simulink model "v01_16r4t11f_ver149bb"  (Simulink 7.9) (continued)
== system v01_16r4t11f_ver149bb == (continued)
      mask init: same as v01_16r4t11f_ver149bb/adcsnap0
      mask StyleString: popup(bram|dram),popup(1|2|3|4),popup(200|250|266|300|333),edit,popup(8|16|32|64|128),checkbox,checkbox,checkbox,checkbox
      mask CallbackString: snapshot_callback();||||||||
  sg : Subsystem
  speader1 : Subsystem  mask{spead_msw=64, spead_lsw=40, num_items=6, num_payloads=2}
      mask prompts: num_items "Number of items (+5)"; num_payloads "number of payloads"
      mask help:
      | The size of the heap and payload are in Bytes.
      | If there is one packet per heap then payload_offset is 0.
      | All the input must be not longer than 40 bits.
  spectrum_cntr : xbsIndex_r4/Counter  cnt_type=Free Running  cnt_to=Inf  operation=Up  start_count=0  cnt_by_val=1  arith_type=Unsigned  n_bits=40  bin_pt=0  load_pin=off  rst=on  en=on  explicit_period=on  period=1  dbl_ovrd=off  use_behavioral_HDL=off  implementation=Fabric  block_type=counter
      settings: block_version=11.4
  ssg : Subsystem
  status : xps_library/software register  Tag=xps:sw_reg  io_dir=To Processor  arith_type=Unsigned  bitwidth=32  bin_pt=0  sample_period=1  gw_name=v01_16r4t11f_ver148bb_status_user_data_in
  stokesout : Subsystem  AncestorBlock=casper_library_scopes/snapshot  mask(snapshot){storage=bram, dram_dimm=1, dram_clock=200, nsamples=10, data_width=32, offset=off, circap=off, value=off, use_dsp48=on}
      mask prompts: storage "Storage medium"; dram_dimm "DRAM dimm"; dram_clock "DRAM clock rate"; nsamples "Number of Samples (2^?)"; data_width "Data width"; offset "Start delay support"; circap "Circular capture support"; value "Extra value capture support"; use_dsp48 "Use DSP48s to implement counters"
      mask help:
      | Standard snap block, but with the following optional extras;
      |  1) Optionally delay the fabric trigger by a user-specified number of valids.
      |  2) Capture continuously after a trigger until a stop pulse is received. Data is captured into BRAM continuously, with old data being overwritten until a stop command is received. Then stop capturing when memory is filled. This enables you to get some data before and some data after the stop pulse. Use the 'tr_en_cnt' register to determine where you are in your vector. 'Addr' register's MSb now indicates 'busy capturing'.
      |  3) Optionally capture a value on an input port to a register at the same time as the first data sample.
      |  4) Implement counters using DSP48Es to save resources.
      mask init: same as v01_16r4t11f_ver149bb/adcsnap0
      mask StyleString: popup(bram|dram),popup(1|2|3|4),popup(200|250|266|300|333),edit,popup(8|16|32|64|128),checkbox,checkbox,checkbox,checkbox
      mask CallbackString: snapshot_callback();||||||||
  sync_delay : casper_library_delays/sync_delay  DelayLen=760*128
  time_cntr : xbsIndex_r4/Counter  cnt_type=Free Running  cnt_to=Inf  operation=Up  start_count=0  cnt_by_val=1  arith_type=Unsigned  n_bits=40  bin_pt=0  load_pin=off  rst=on  en=off  explicit_period=on  period=1  dbl_ovrd=off  use_behavioral_HDL=off  implementation=Fabric  block_type=counter
      settings: block_version=11.4
  timesel : xps_library/software register  Tag=xps:sw_reg  io_dir=From Processor  arith_type=Unsigned  bitwidth=32  bin_pt=0  sample_period=1  gw_name=v01_16r4t11f_ver148bb_timesel_user_data_out
  timesnap : Subsystem  AncestorBlock=casper_library_scopes/snapshot  mask(snapshot){storage=bram, dram_dimm=1, dram_clock=200, nsamples=10, data_width=64, offset=off, circap=off, value=off, use_dsp48=on}
      mask prompts: storage "Storage medium"; dram_dimm "DRAM dimm"; dram_clock "DRAM clock rate"; nsamples "Number of Samples (2^?)"; data_width "Data width"; offset "Start delay support"; circap "Circular capture support"; value "Extra value capture support"; use_dsp48 "Use DSP48s to implement counters"
      mask help:
      | Standard snap block, but with the following optional extras;
      |  1) Optionally delay the fabric trigger by a user-specified number of valids.
      |  2) Capture continuously after a trigger until a stop pulse is received. Data is captured into BRAM continuously, with old data being overwritten until a stop command is received. Then stop capturing when memory is filled. This enables you to get some data before and some data after the stop pulse. Use the 'tr_en_cnt' register to determine where you are in your vector. 'Addr' register's MSb now indicates 'busy capturing'.
      |  3) Optionally capture a value on an input port to a register at the same time as the first data sample.
      |  4) Implement counters using DSP48Es to save resources.
      mask init: same as v01_16r4t11f_ver149bb/adcsnap0
      mask StyleString: popup(bram|dram),popup(1|2|3|4),popup(200|250|266|300|333),edit,popup(8|16|32|64|128),checkbox,checkbox,checkbox,checkbox
      mask CallbackString: snapshot_callback();||||||||
  vacc0 : Subsystem  AncestorBlock=gavrt_library/vacc  mask(vacc){veclen=7, n_inputs=4, max_accum=10, arith_type=1, in_bit_width=32, in_bin_pt=0, out_bit_width=42, out_bin_pt=0, register_din=1, add_latency=2, bram_latency=2, mux_latency=0, use_dsp48=1}
      mask prompts: veclen "Vector Length (2^?)"; n_inputs "Number of Simultaneous Vectors"; max_accum "Maximum # Accumulations (2^?)"; arith_type "Arithmetic Type (0=Unsigned, 1=Signed)"; in_bit_width "Input Bit Width"; in_bin_pt "Input Binary Point"; out_bit_width "Output Bit Width"; out_bin_pt "Output Binary Point"; register_din "Register Input Data  (1 = Enable Register Retiming on input delay)"; add_latency "Add Latency"; bram_latency "BRAM Latency"; mux_latency "Mux Latency"; use_dsp48 "Use DSP48"
      mask help:
      | Sync is expected the clock before the first data to be accumulated.
      | Use the Cram block to feed din for multiple simultaneous inputs.
      | acc_len is the number of vectors to sum.
      | valid is high while a valid accumulation is being clocked out on dout.
      | If only one sync pulse is provided, valid accumulations will repeatedly appear on dout as they become available.
      | Undesired behavior may occur if acc_len is changed without a new sync pulse.
      | Note: The output is an unsigned concatenation of the output streams. Thus, even with only one stream, the output must be recast before using.
      | Register Input Data controls the "Enable Register Retiming" parameter of the delays on 'din'.
      mask init:
      | config.toplevel = gcb;
      | config.source = str2func('vacc_init_xblock');
      | xBlock( config, {...
      |     'veclen', veclen, ...
      |     'n_inputs', n_inputs, ...
      |     'max_accum', max_accum, ...
      |     'arith_type', arith_type, ...
      |     'in_bit_width', in_bit_width, ...
      |     'in_bin_pt', in_bin_pt, ...
      |     'out_bit_width', out_bit_width, ...
      |     'out_bin_pt', out_bin_pt, ...
      |     'register_din', register_din, ...
      |     'add_latency', add_latency, ...
      |     'bram_latency', bram_latency, ...
      |     'mux_latency', mux_latency, ...
      |     'use_dsp48', use_dsp48, ...
      | });
      settings: AttributesFormatString=|
      | vector length=32, inputs=4
      | max accumulations=2^5
  vacc1 : Subsystem  AncestorBlock=gavrt_library/vacc  mask(vacc){veclen=7, n_inputs=4, max_accum=10, arith_type=1, in_bit_width=32, in_bin_pt=0, out_bit_width=42, out_bin_pt=0, register_din=1, add_latency=2, bram_latency=2, mux_latency=0, use_dsp48=1}
      mask prompts: veclen "Vector Length (2^?)"; n_inputs "Number of Simultaneous Vectors"; max_accum "Maximum # Accumulations (2^?)"; arith_type "Arithmetic Type (0=Unsigned, 1=Signed)"; in_bit_width "Input Bit Width"; in_bin_pt "Input Binary Point"; out_bit_width "Output Bit Width"; out_bin_pt "Output Binary Point"; register_din "Register Input Data  (1 = Enable Register Retiming on input delay)"; add_latency "Add Latency"; bram_latency "BRAM Latency"; mux_latency "Mux Latency"; use_dsp48 "Use DSP48"
      mask help:
      | Sync is expected the clock before the first data to be accumulated.
      | Use the Cram block to feed din for multiple simultaneous inputs.
      | acc_len is the number of vectors to sum.
      | valid is high while a valid accumulation is being clocked out on dout.
      | If only one sync pulse is provided, valid accumulations will repeatedly appear on dout as they become available.
      | Undesired behavior may occur if acc_len is changed without a new sync pulse.
      | Note: The output is an unsigned concatenation of the output streams. Thus, even with only one stream, the output must be recast before using.
      | Register Input Data controls the "Enable Register Retiming" parameter of the delays on 'din'.
      mask init: same as v01_16r4t11f_ver149bb/vacc0
      settings: AttributesFormatString=|
      | vector length=32, inputs=4
      | max accumulations=2^5
  vacc2 : Subsystem  AncestorBlock=gavrt_library/vacc  mask(vacc){veclen=7, n_inputs=4, max_accum=10, arith_type=1, in_bit_width=32, in_bin_pt=0, out_bit_width=42, out_bin_pt=0, register_din=1, add_latency=2, bram_latency=2, mux_latency=0, use_dsp48=1}
      mask prompts: veclen "Vector Length (2^?)"; n_inputs "Number of Simultaneous Vectors"; max_accum "Maximum # Accumulations (2^?)"; arith_type "Arithmetic Type (0=Unsigned, 1=Signed)"; in_bit_width "Input Bit Width"; in_bin_pt "Input Binary Point"; out_bit_width "Output Bit Width"; out_bin_pt "Output Binary Point"; register_din "Register Input Data  (1 = Enable Register Retiming on input delay)"; add_latency "Add Latency"; bram_latency "BRAM Latency"; mux_latency "Mux Latency"; use_dsp48 "Use DSP48"
      mask help:
      | Sync is expected the clock before the first data to be accumulated.
      | Use the Cram block to feed din for multiple simultaneous inputs.
      | acc_len is the number of vectors to sum.
      | valid is high while a valid accumulation is being clocked out on dout.
      | If only one sync pulse is provided, valid accumulations will repeatedly appear on dout as they become available.
      | Undesired behavior may occur if acc_len is changed without a new sync pulse.
      | Note: The output is an unsigned concatenation of the output streams. Thus, even with only one stream, the output must be recast before using.
      | Register Input Data controls the "Enable Register Retiming" parameter of the delays on 'din'.
      mask init: same as v01_16r4t11f_ver149bb/vacc0
      settings: AttributesFormatString=|
      | vector length=32, inputs=4
      | max accumulations=2^5
  vacc3 : Subsystem  AncestorBlock=gavrt_library/vacc  mask(vacc){veclen=7, n_inputs=4, max_accum=10, arith_type=1, in_bit_width=32, in_bin_pt=0, out_bit_width=42, out_bin_pt=0, register_din=1, add_latency=2, bram_latency=2, mux_latency=0, use_dsp48=1}
      mask prompts: veclen "Vector Length (2^?)"; n_inputs "Number of Simultaneous Vectors"; max_accum "Maximum # Accumulations (2^?)"; arith_type "Arithmetic Type (0=Unsigned, 1=Signed)"; in_bit_width "Input Bit Width"; in_bin_pt "Input Binary Point"; out_bit_width "Output Bit Width"; out_bin_pt "Output Binary Point"; register_din "Register Input Data  (1 = Enable Register Retiming on input delay)"; add_latency "Add Latency"; bram_latency "BRAM Latency"; mux_latency "Mux Latency"; use_dsp48 "Use DSP48"
      mask help:
      | Sync is expected the clock before the first data to be accumulated.
      | Use the Cram block to feed din for multiple simultaneous inputs.
      | acc_len is the number of vectors to sum.
      | valid is high while a valid accumulation is being clocked out on dout.
      | If only one sync pulse is provided, valid accumulations will repeatedly appear on dout as they become available.
      | Undesired behavior may occur if acc_len is changed without a new sync pulse.
      | Note: The output is an unsigned concatenation of the output streams. Thus, even with only one stream, the output must be recast before using.
      | Register Input Data controls the "Enable Register Retiming" parameter of the delays on 'din'.
      mask init: same as v01_16r4t11f_ver149bb/vacc0
      settings: AttributesFormatString=|
      | vector length=32, inputs=4
      | max accumulations=2^5
  vacc4 : Subsystem  AncestorBlock=gavrt_library/vacc  mask(vacc){veclen=7, n_inputs=4, max_accum=10, arith_type=1, in_bit_width=32, in_bin_pt=0, out_bit_width=42, out_bin_pt=0, register_din=1, add_latency=2, bram_latency=2, mux_latency=0, use_dsp48=1}
      mask prompts: veclen "Vector Length (2^?)"; n_inputs "Number of Simultaneous Vectors"; max_accum "Maximum # Accumulations (2^?)"; arith_type "Arithmetic Type (0=Unsigned, 1=Signed)"; in_bit_width "Input Bit Width"; in_bin_pt "Input Binary Point"; out_bit_width "Output Bit Width"; out_bin_pt "Output Binary Point"; register_din "Register Input Data  (1 = Enable Register Retiming on input delay)"; add_latency "Add Latency"; bram_latency "BRAM Latency"; mux_latency "Mux Latency"; use_dsp48 "Use DSP48"
      mask help:
      | Sync is expected the clock before the first data to be accumulated.
      | Use the Cram block to feed din for multiple simultaneous inputs.
      | acc_len is the number of vectors to sum.
      | valid is high while a valid accumulation is being clocked out on dout.
      | If only one sync pulse is provided, valid accumulations will repeatedly appear on dout as they become available.
      | Undesired behavior may occur if acc_len is changed without a new sync pulse.
      | Note: The output is an unsigned concatenation of the output streams. Thus, even with only one stream, the output must be recast before using.
      | Register Input Data controls the "Enable Register Retiming" parameter of the delays on 'din'.
      mask init: same as v01_16r4t11f_ver149bb/vacc0
      settings: AttributesFormatString=|
      | vector length=32, inputs=4
      | max accumulations=2^5
  vacc5 : Subsystem  AncestorBlock=gavrt_library/vacc  mask(vacc){veclen=7, n_inputs=4, max_accum=10, arith_type=1, in_bit_width=32, in_bin_pt=0, out_bit_width=42, out_bin_pt=0, register_din=1, add_latency=2, bram_latency=2, mux_latency=0, use_dsp48=1}
      mask prompts: veclen "Vector Length (2^?)"; n_inputs "Number of Simultaneous Vectors"; max_accum "Maximum # Accumulations (2^?)"; arith_type "Arithmetic Type (0=Unsigned, 1=Signed)"; in_bit_width "Input Bit Width"; in_bin_pt "Input Binary Point"; out_bit_width "Output Bit Width"; out_bin_pt "Output Binary Point"; register_din "Register Input Data  (1 = Enable Register Retiming on input delay)"; add_latency "Add Latency"; bram_latency "BRAM Latency"; mux_latency "Mux Latency"; use_dsp48 "Use DSP48"
      mask help:
      | Sync is expected the clock before the first data to be accumulated.
      | Use the Cram block to feed din for multiple simultaneous inputs.
      | acc_len is the number of vectors to sum.
      | valid is high while a valid accumulation is being clocked out on dout.
      | If only one sync pulse is provided, valid accumulations will repeatedly appear on dout as they become available.
      | Undesired behavior may occur if acc_len is changed without a new sync pulse.
      | Note: The output is an unsigned concatenation of the output streams. Thus, even with only one stream, the output must be recast before using.
      | Register Input Data controls the "Enable Register Retiming" parameter of the delays on 'din'.
      mask init: same as v01_16r4t11f_ver149bb/vacc0
      settings: AttributesFormatString=|
      | vector length=32, inputs=4
      | max accumulations=2^5
  vacc6 : Subsystem  AncestorBlock=gavrt_library/vacc  mask(vacc){veclen=7, n_inputs=4, max_accum=10, arith_type=1, in_bit_width=32, in_bin_pt=0, out_bit_width=42, out_bin_pt=0, register_din=1, add_latency=2, bram_latency=2, mux_latency=0, use_dsp48=1}
      mask prompts: veclen "Vector Length (2^?)"; n_inputs "Number of Simultaneous Vectors"; max_accum "Maximum # Accumulations (2^?)"; arith_type "Arithmetic Type (0=Unsigned, 1=Signed)"; in_bit_width "Input Bit Width"; in_bin_pt "Input Binary Point"; out_bit_width "Output Bit Width"; out_bin_pt "Output Binary Point"; register_din "Register Input Data  (1 = Enable Register Retiming on input delay)"; add_latency "Add Latency"; bram_latency "BRAM Latency"; mux_latency "Mux Latency"; use_dsp48 "Use DSP48"
      mask help:
      | Sync is expected the clock before the first data to be accumulated.
      | Use the Cram block to feed din for multiple simultaneous inputs.
      | acc_len is the number of vectors to sum.
      | valid is high while a valid accumulation is being clocked out on dout.
      | If only one sync pulse is provided, valid accumulations will repeatedly appear on dout as they become available.
      | Undesired behavior may occur if acc_len is changed without a new sync pulse.
      | Note: The output is an unsigned concatenation of the output streams. Thus, even with only one stream, the output must be recast before using.
      | Register Input Data controls the "Enable Register Retiming" parameter of the delays on 'din'.
      mask init: same as v01_16r4t11f_ver149bb/vacc0
      settings: AttributesFormatString=|
      | vector length=32, inputs=4
      | max accumulations=2^5
  vacc7 : Subsystem  AncestorBlock=gavrt_library/vacc  mask(vacc){veclen=7, n_inputs=4, max_accum=10, arith_type=1, in_bit_width=32, in_bin_pt=0, out_bit_width=42, out_bin_pt=0, register_din=1, add_latency=2, bram_latency=2, mux_latency=0, use_dsp48=1}
      mask prompts: veclen "Vector Length (2^?)"; n_inputs "Number of Simultaneous Vectors"; max_accum "Maximum # Accumulations (2^?)"; arith_type "Arithmetic Type (0=Unsigned, 1=Signed)"; in_bit_width "Input Bit Width"; in_bin_pt "Input Binary Point"; out_bit_width "Output Bit Width"; out_bin_pt "Output Binary Point"; register_din "Register Input Data  (1 = Enable Register Retiming on input delay)"; add_latency "Add Latency"; bram_latency "BRAM Latency"; mux_latency "Mux Latency"; use_dsp48 "Use DSP48"
      mask help:
      | Sync is expected the clock before the first data to be accumulated.
      | Use the Cram block to feed din for multiple simultaneous inputs.
      | acc_len is the number of vectors to sum.
      | valid is high while a valid accumulation is being clocked out on dout.
      | If only one sync pulse is provided, valid accumulations will repeatedly appear on dout as they become available.
      | Undesired behavior may occur if acc_len is changed without a new sync pulse.
      | Note: The output is an unsigned concatenation of the output streams. Thus, even with only one stream, the output must be recast before using.
      | Register Input Data controls the "Enable Register Retiming" parameter of the delays on 'din'.
      mask init: same as v01_16r4t11f_ver149bb/vacc0
      settings: AttributesFormatString=|
      | vector length=32, inputs=4
      | max accumulations=2^5
  vaccout : Subsystem  AncestorBlock=casper_library_scopes/snapshot  mask(snapshot){storage=bram, dram_dimm=1, dram_clock=200, nsamples=10, data_width=32, offset=off, circap=off, value=off, use_dsp48=on}
      mask prompts: storage "Storage medium"; dram_dimm "DRAM dimm"; dram_clock "DRAM clock rate"; nsamples "Number of Samples (2^?)"; data_width "Data width"; offset "Start delay support"; circap "Circular capture support"; value "Extra value capture support"; use_dsp48 "Use DSP48s to implement counters"
      mask help:
      | Standard snap block, but with the following optional extras;
      |  1) Optionally delay the fabric trigger by a user-specified number of valids.
      |  2) Capture continuously after a trigger until a stop pulse is received. Data is captured into BRAM continuously, with old data being overwritten until a stop command is received. Then stop capturing when memory is filled. This enables you to get some data before and some data after the stop pulse. Use the 'tr_en_cnt' register to determine where you are in your vector. 'Addr' register's MSb now indicates 'busy capturing'.
      |  3) Optionally capture a value on an input port to a register at the same time as the first data sample.
      |  4) Implement counters using DSP48Es to save resources.
      mask init: same as v01_16r4t11f_ver149bb/adcsnap0
      mask StyleString: popup(bram|dram),popup(1|2|3|4),popup(200|250|266|300|333),edit,popup(8|16|32|64|128),checkbox,checkbox,checkbox,checkbox
      mask CallbackString: snapshot_callback();||||||||
  wires:
  manrst/1 -> Register16/1
  Constant19/1 -> manrst/1
  Register16/1 -> Slice2/1, Slice4/1
  Slice2/1 -> Delay26/1
  Register10/1 -> [xout0]
  Register11/1 -> [xout1]
  Register9/1 -> Delay2/1
  Register48/1 -> [yout0]
  Register49/1 -> [yout1]
  Slice10/1 -> edge_detect5/1
  Register44/1 -> Slice10/1
  Constant6/1 -> gbe0/7
  gbe0/10 -> Terminator/1
  gbe0/2 -> Terminator17/1
  gbe0/3 -> Terminator16/1
  gbe0/4 -> Terminator15/1
  gbe0/5 -> [txovf]
  gbe0/6 -> Terminator8/1
  gbe0/7 -> Terminator6/1
  gbe0/8 -> Terminator5/1
  gbe0/9 -> Terminator3/1
  gbe0/11 -> Terminator11/1
  Constant5/1 -> gbe0/8
  Delay21/1 -> gbe0/6
  Delay25/1 -> gbe0/3
  Delay29/1 -> gbe0/2
  Delay30/1 -> gbe0/1
  acc_len/1 -> Delay32/1
  Constant3/1 -> acc_len/1
  Delay32/1 -> [acc_len]
  err_clear_sim1/1 -> dest_ip/1
  dest_ip/1 -> [dest_ip]
  dest_port/1 -> Slice7/1
  err_clear_sim3/1 -> dest_port/1
  Slice7/1 -> [dest_port]
  [tge_rst] -> Delay30/1
  [data_out] -> speader1/1(data_in)
  [dest_ip] -> gbe0/4
  [dest_port] -> gbe0/5
  speader1/3(eof) -> [eof]
  [eof] -> Delay21/1
  Constant18/1 -> speader1/3(heap_size)
  Constant33/1 -> speader1/8(mode)
  Constant34/1 -> speader1/10(payload_offset)
  speader1/2(data_valid) -> [data_valid_spead]
  [data_spead] -> Delay29/1
  [master_rst1] -> time_cntr/1
  Slice5/1 -> [xxout0]
  Slice6/1 -> [yyout0]
  Slice13/1 -> [xyoutre0]
  Slice18/1 -> [xyoutim0]
  Slice19/1 -> [xxout1]
  Slice20/1 -> [yyout1]
  Slice21/1 -> [xyoutre1]
  Slice22/1 -> [xyoutim1]
  [acc_len] -> Convert/1
  Constant24/1 -> speader1/4(Packet_length)
  [master_rst1] -> spectrum_cntr/1
  [eoh] -> spectrum_cntr/2
  [spead_rst] -> speader1/11(Reset)
  Convert/1 -> speader1/7(integration size)
  speader1/1(data_out) -> [data_spead]
  [fftshift] -> Delay5/1
  Register1/1 -> [xout2]
  Register2/1 -> [xout3]
  Register12/1 -> [yout2]
  Register13/1 -> [yout3]
  Slice29/1 -> [xxout2]
  Slice30/1 -> [yyout2]
  Slice14/1 -> [xyoutre2]
  Slice15/1 -> [xyoutim2]
  Slice25/1 -> [xxout3]
  Slice26/1 -> [yyout3]
  Slice27/1 -> [xyoutre3]
  Slice28/1 -> [xyoutim3]
  spectrum_cntr/1 -> [spec_cntr]
  time_cntr/1 -> [time_cntr]
  [time_cntr] -> speader1/5(time_stamp)
  [spec_cntr] -> speader1/6(spectrum counter)
  cram4/1(Out) -> [din]
  speader1/4(eoh) -> [eoh]
  reshaper/2(sel) -> Terminator10/1
  reshaper/3(header_valid_out) -> Terminator13/1
  [din] -> Delay13/1
  Delay10/1 -> Delay11/1, edge_detect4/1(in)
  Delay13/1 -> Delay12/1
  Delay12/1 -> reshaper/3(din)
  Delay11/1 -> reshaper/2(valid)
  reshaper/5(st) -> [reshape_state]
  Delay14/1 -> [data_out], Slice41/1
  Delay15/1 -> [data_valid], rshpout/3(trig), Delay64/1
  reshaper/1(dout) -> Delay14/1
  reshaper/4(data_valid_out) -> Delay15/1
  Constant30/1 -> Register19/1
  Register18/1 -> Inverter/1
  Inverter/1 -> Register19/2, [spead_rst]  "ppsready"
  Constant31/1 -> Register18/1
  arm/1 -> Register20/1
  Constant12/1 -> arm/1
  Register20/1 -> Slice3/1
  Slice3/1 -> [arm]
  [arm] -> Register18/2
  [master_rst1] -> Register18/3
  [sync_out] -> sync_delay/1
  Register19/1 -> [data_enable]
  [valid] -> Logical/1
  [data_enable] -> Logical/2
  Logical/1 -> Delay10/1
  Delay4/1 -> speader1/9(status_bits)
  [fftshift] -> Delay6/1
  [adc_sync] -> sg/1(pps)
  gbe0/1 -> Terminator9/1, [ledup]
  Slice4/1 -> [auto_reset_enable]
  [arm] -> edge_detect2/1
  edge_detect2/1 -> Delay60/1
  Logical2/1 -> [tge_rst]
  Delay26/1 -> Logical2/1
  Logical3/1 -> Logical2/3
  [auto_reset_enable] -> Logical3/1
  [txovf] -> edge_detect1/1
  edge_detect1/1 -> Logical3/2
  [master_rst1] -> Counter1/1
  Counter1/1 -> Slice8/1
  Slice8/1 -> edge_detect3/1(in)
  edge_detect3/1(out) -> [spec_tick]
  Constant10/1 -> bus_create2/1(in1)
  [status_bits] -> bus_create2/2(in2)
  bus_create2/1(bus_out) -> Delay4/1
  vacc0/2(dout_1_1) -> Slice1/1
  vacc0/3(dout_2_1) -> Slice11/1
  vacc1/2(dout_1_1) -> Slice17/1
  vacc1/3(dout_2_1) -> Slice23/1
  vacc2/2(dout_1_1) -> Slice32/1
  vacc2/3(dout_2_1) -> Slice33/1
  vacc3/2(dout_1_1) -> Slice36/1
  vacc3/3(dout_2_1) -> Slice37/1
  vacc0/4(dout_3_1) -> Slice12/1
  vacc0/5(dout_4_1) -> Slice16/1
  vacc2/4(dout_3_1) -> Slice34/1
  vacc2/5(dout_4_1) -> Slice35/1
  vacc3/4(dout_3_1) -> Slice38/1
  vacc3/5(dout_4_1) -> Slice39/1
  [sync_out] -> vacc0/1(sync)
  [acc_len] -> vacc0/2(acc_len)
  [acc_len] -> vacc3/2(acc_len)
  [sync_out] -> vacc2/1(sync)
  [sync_out] -> vacc3/1(sync)
  [acc_len] -> vacc2/2(acc_len)
  vacc1/1(valid) -> [valid]
  vacc2/1(valid) -> Terminator4/1
  vacc3/1(valid) -> Terminator7/1
  vacc1/4(dout_3_1) -> Slice24/1
  vacc1/5(dout_4_1) -> Slice31/1
  [sync_out] -> vacc1/1(sync)
  [acc_len] -> vacc1/2(acc_len)
  [xxout0] -> reinterp_vacc/1
  [yyout0] -> reinterp_vacc1/1
  [xyoutre0] -> reinterp_vacc2/1
  [xyoutim0] -> reinterp_vacc3/1
  [xxout1] -> reinterp_vacc4/1
  [yyout1] -> reinterp_vacc5/1
  [xyoutre1] -> reinterp_vacc6/1
  [xyoutim1] -> reinterp_vacc7/1
  [xxout2] -> reinterp_vacc8/1
  [yyout2] -> reinterp_vacc9/1
  [xyoutre2] -> reinterp_vacc10/1
  [xyoutim2] -> reinterp_vacc11/1
  [xxout3] -> reinterp_vacc12/1
  [yyout3] -> reinterp_vacc13/1
  [xyoutre3] -> reinterp_vacc14/1
  [xyoutim3] -> reinterp_vacc15/1
  vacc0/1(valid) -> Terminator1/1
  sg/1(sync_out) -> [sync_gen_0]
  sg/2(pps_out) -> [master_rst1]
  Slice1/1 -> [xx_vacc0]
  Slice11/1 -> [yy_vacc0]
  Slice12/1 -> [xy_vaccre0]
  Slice16/1 -> [xy_vaccim0]
  Slice17/1 -> [xx_vacc1]
  Slice23/1 -> [yy_vacc1]
  Slice24/1 -> [xy_vaccre1]
  Slice31/1 -> [xy_vaccim1]
  Slice32/1 -> [xx_vacc2]
  Slice33/1 -> [yy_vacc2]
  Slice34/1 -> [xy_vaccre2]
  Slice35/1 -> [xy_vaccim2]
  Slice36/1 -> [xx_vacc3]
  Slice37/1 -> [yy_vacc3]
  Slice38/1 -> [xy_vaccre3]
  Slice39/1 -> [xy_vaccim3]
  reinterp_vacc/1 -> vacc0/3(din_1_1)
  reinterp_vacc1/1 -> vacc0/4(din_2_1)
  reinterp_vacc2/1 -> vacc0/5(din_3_1)
  reinterp_vacc3/1 -> vacc0/6(din_4_1)
  reinterp_vacc4/1 -> vacc1/3(din_1_1)
  reinterp_vacc5/1 -> vacc1/4(din_2_1)
  reinterp_vacc6/1 -> vacc1/5(din_3_1)
  reinterp_vacc7/1 -> vacc1/6(din_4_1)
  reinterp_vacc8/1 -> vacc2/3(din_1_1)
  reinterp_vacc9/1 -> vacc2/4(din_2_1)
  reinterp_vacc10/1 -> vacc2/5(din_3_1)
  reinterp_vacc11/1 -> vacc2/6(din_4_1)
  reinterp_vacc12/1 -> vacc3/3(din_1_1)
  reinterp_vacc13/1 -> vacc3/4(din_2_1)
  reinterp_vacc14/1 -> vacc3/5(din_3_1)
  reinterp_vacc15/1 -> vacc3/6(din_4_1)
  Constant14/1 -> raw_adc_trig/1
  [spead_rst] -> sg/2(pps_en)
  ssg/1(statusbits) -> [status_bits]
  Subsystem1/1(Out1) -> [adc_a]
  Subsystem2/1(Out1) -> [adc_b]
  asiaa_adc5g1/17(sync_out) -> Register44/1
  asiaa_adc5g2/17(sync_out) -> Terminator43/1
  asiaa_adc5g1/1(a0) -> Delay7/1
  asiaa_adc5g1/2(a1) -> Delay9/1
  asiaa_adc5g1/3(a2) -> Delay18/1
  asiaa_adc5g1/4(a3) -> Delay20/1
  asiaa_adc5g1/5(a4) -> Delay22/1
  asiaa_adc5g1/6(a5) -> Delay19/1
  asiaa_adc5g1/7(a6) -> Delay31/1
  asiaa_adc5g1/8(a7) -> Delay33/1
  asiaa_adc5g1/9(a8) -> Delay23/1
  asiaa_adc5g1/10(a9) -> Delay27/1
  asiaa_adc5g1/11(a10) -> Delay28/1
  asiaa_adc5g1/12(a11) -> Delay24/1
  asiaa_adc5g1/13(a12) -> Delay34/1
  asiaa_adc5g1/14(a13) -> Delay36/1
  asiaa_adc5g1/15(a14) -> Delay37/1
  asiaa_adc5g1/16(a15) -> Delay35/1
  asiaa_adc5g2/1(a0) -> Delay56/1
  asiaa_adc5g2/2(a1) -> Delay57/1
  asiaa_adc5g2/3(a2) -> Delay42/1
  asiaa_adc5g2/4(a3) -> Delay44/1
  asiaa_adc5g2/5(a4) -> Delay45/1
  asiaa_adc5g2/6(a5) -> Delay43/1
  asiaa_adc5g2/7(a6) -> Delay50/1
  asiaa_adc5g2/8(a7) -> Delay51/1
  asiaa_adc5g2/9(a8) -> Delay46/1
  asiaa_adc5g2/10(a9) -> Delay48/1
  asiaa_adc5g2/11(a10) -> Delay49/1
  asiaa_adc5g2/12(a11) -> Delay47/1
  asiaa_adc5g2/13(a12) -> Delay52/1
  asiaa_adc5g2/14(a13) -> Delay54/1
  asiaa_adc5g2/15(a14) -> Delay55/1
  asiaa_adc5g2/16(a15) -> Delay53/1
  Delay7/1 -> Subsystem1/1(In1)
  Delay9/1 -> Subsystem1/2(In2)
  Delay18/1 -> Subsystem1/3(In3)
  Delay20/1 -> Subsystem1/4(In4)
  Delay22/1 -> Subsystem1/5(In5)
  Delay19/1 -> Subsystem1/6(In6)
  Delay31/1 -> Subsystem1/7(In7)
  Delay33/1 -> Subsystem1/8(In8)
  Delay23/1 -> Subsystem1/9(In9)
  Delay27/1 -> Subsystem1/10(In10)
  Delay28/1 -> Subsystem1/11(In11)
  Delay24/1 -> Subsystem1/12(In12)
  Delay34/1 -> Subsystem1/13(In13)
  Delay36/1 -> Subsystem1/14(In14)
  Delay37/1 -> Subsystem1/15(In15)
  Delay35/1 -> Subsystem1/16(In16)
  Delay56/1 -> Subsystem2/1(In1)
  Delay57/1 -> Subsystem2/2(In2)
  Delay42/1 -> Subsystem2/3(In3)
  Delay44/1 -> Subsystem2/4(In4)
  Delay45/1 -> Subsystem2/5(In5)
  Delay43/1 -> Subsystem2/6(In6)
  Delay50/1 -> Subsystem2/7(In7)
  Delay51/1 -> Subsystem2/8(In8)
  Delay46/1 -> Subsystem2/9(In9)
  Delay48/1 -> Subsystem2/10(In10)
  Delay49/1 -> Subsystem2/11(In11)
  Delay47/1 -> Subsystem2/12(In12)
  Delay52/1 -> Subsystem2/13(In13)
  Delay54/1 -> Subsystem2/14(In14)
  Delay55/1 -> Subsystem2/15(In15)
  Delay53/1 -> Subsystem2/16(In16)
  [sync_gen_0] -> pfb0/1
  [sync_gen_0] -> pfb1/1
  Delay5/1 -> fft0/2
  Delay6/1 -> fft1/2
  fft0/1 -> Register9/1
  Register4/1 -> [xout4]
  Register5/1 -> [xout5]
  Register3/1 -> [xout6]
  Register6/1 -> [xout7]
  fft0/8 -> Register3/1
  fft0/9 -> Register6/1
  fft0/7 -> Register5/1
  fft0/6 -> Register4/1
  fft0/5 -> Register2/1
  fft0/4 -> Register1/1
  fft0/3 -> Register11/1
  fft0/2 -> Register10/1
  fft0/10 -> Terminator12/1
  fft1/1 -> Terminator2/1
  Register15/1 -> [yout5]
  Register7/1 -> [yout6]
  Register8/1 -> [yout7]
  fft1/2 -> Register48/1
  fft1/3 -> Register49/1
  fft1/4 -> Register12/1
  Register14/1 -> [yout4]
  [xout4] -> Stokes/9(x4)
  [yout4] -> Stokes/10(y4)
  [xout5] -> Stokes/11(x5)
  [yout5] -> Stokes/12(y5)
  [xout6] -> Stokes/13(x6)
  [yout6] -> Stokes/14(y6)
  [xout7] -> Stokes/15(x7)
  [yout7] -> Stokes/16(y7)
  [yout3] -> Stokes/8(y3)
  [xout3] -> Stokes/7(x3)
  [yout2] -> Stokes/6(y2)
  [xout2] -> Stokes/5(x2)
  [yout1] -> Stokes/4(y1)
  [xout1] -> Stokes/3(x1)
  [yout0] -> Stokes/2(y0)
  [xout0] -> Stokes/1(x0)
  Slice59/1 -> [yyout4]
  Slice44/1 -> [xyoutre4]
  Slice47/1 -> [xyoutim4]
  Slice48/1 -> [xxout5]
  Slice49/1 -> [yyout5]
  Slice50/1 -> [xyoutre5]
  Slice51/1 -> [xyoutim5]
  Slice56/1 -> [xxout6]
  Slice57/1 -> [yyout6]
  Slice45/1 -> [xyoutre6]
  Slice46/1 -> [xyoutim6]
  Slice52/1 -> [xxout7]
  Slice53/1 -> [yyout7]
  Slice54/1 -> [xyoutre7]
  Slice55/1 -> [xyoutim7]
  Slice58/1 -> [xxout4]
  Stokes/1(x0x0*) -> Slice5/1
  Stokes/2(y0y0*) -> Slice6/1
  Stokes/3(x0y0*_re) -> Slice13/1
  Stokes/4(x0y0*_im) -> Slice18/1
  Stokes/5(x1x1*) -> Slice19/1
  Stokes/6(y1y1*) -> Slice20/1
  Stokes/7(x1y1*_re) -> Slice21/1
  Stokes/8(x1y1*_im) -> Slice22/1
  Stokes/9(x2x2*) -> Slice29/1
  Stokes/10(y2y2*) -> Slice30/1
  Stokes/11(x2y2*_re) -> Slice14/1
  Stokes/12(x2y2*_im) -> Slice15/1
  Stokes/13(x3x3*) -> Slice25/1
  Stokes/14(y3y3*) -> Slice26/1
  Stokes/15(x3y3*_re) -> Slice27/1
  Stokes/16(x3y3*_im) -> Slice28/1
  Stokes/17(x4x4*) -> Slice58/1
  Stokes/18(y4y4*) -> Slice59/1
  Stokes/19(x4y4*_re) -> Slice44/1
  Stokes/20(x4y4*_im) -> Slice47/1
  Stokes/21(x5x5*) -> Slice48/1
  Stokes/22(y5y5*) -> Slice49/1
  Stokes/23(x5y5*_re) -> Slice50/1
  Stokes/24(x5y5*_im) -> Slice51/1
  Stokes/25(x6x6*) -> Slice56/1
  Stokes/26(y6y6*) -> Slice57/1
  Stokes/27(x6y6*_re) -> Slice45/1
  Stokes/28(x6y6*_im) -> Slice46/1
  Stokes/29(x7x7*) -> Slice52/1
  Stokes/30(y7y7*) -> Slice53/1
  Stokes/31(x7y7*_re) -> Slice54/1
  Stokes/32(x7y7*_im) -> Slice55/1
  vacc4/2(dout_1_1) -> Slice60/1
  vacc4/3(dout_2_1) -> Slice61/1
  vacc5/2(dout_1_1) -> Slice64/1
  vacc5/3(dout_2_1) -> Slice65/1
  vacc4/4(dout_3_1) -> Slice62/1
  vacc4/5(dout_4_1) -> Slice63/1
  vacc5/4(dout_3_1) -> Slice66/1
  vacc5/5(dout_4_1) -> Slice67/1
  [acc_len] -> vacc5/2(acc_len)
  [sync_out] -> vacc4/1(sync)
  [sync_out] -> vacc5/1(sync)
  [acc_len] -> vacc4/2(acc_len)
  vacc4/1(valid) -> Terminator14/1
  vacc5/1(valid) -> Terminator18/1
  [xxout6] -> reinterp_vacc22/1
  [yyout6] -> reinterp_vacc23/1
  [xyoutre6] -> reinterp_vacc16/1
  [xyoutim6] -> reinterp_vacc17/1
  [xxout7] -> reinterp_vacc18/1
  [yyout7] -> reinterp_vacc19/1
  [xyoutre7] -> reinterp_vacc20/1
  [xyoutim7] -> reinterp_vacc21/1
  Slice60/1 -> [xx_vacc6]
  Slice61/1 -> [yy_vacc6]
  Slice62/1 -> [xy_vaccre6]
  Slice63/1 -> [xy_vaccim6]
  Slice64/1 -> [xx_vacc7]
  Slice65/1 -> [yy_vacc7]
  Slice66/1 -> [xy_vaccre7]
  Slice67/1 -> [xy_vaccim7]
  reinterp_vacc22/1 -> vacc4/3(din_1_1)
  reinterp_vacc23/1 -> vacc4/4(din_2_1)
  reinterp_vacc16/1 -> vacc4/5(din_3_1)
  reinterp_vacc17/1 -> vacc4/6(din_4_1)
  reinterp_vacc18/1 -> vacc5/3(din_1_1)
  reinterp_vacc19/1 -> vacc5/4(din_2_1)
  reinterp_vacc20/1 -> vacc5/5(din_3_1)
  reinterp_vacc21/1 -> vacc5/6(din_4_1)
  vacc6/2(dout_1_1) -> Slice68/1
  vacc6/3(dout_2_1) -> Slice69/1
  vacc7/2(dout_1_1) -> Slice72/1
  vacc7/3(dout_2_1) -> Slice73/1
  vacc6/4(dout_3_1) -> Slice70/1
  vacc6/5(dout_4_1) -> Slice71/1
  [sync_out] -> vacc6/1(sync)
  [acc_len] -> vacc6/2(acc_len)
  vacc7/1(valid) -> Terminator20/1
  vacc7/4(dout_3_1) -> Slice74/1
  vacc7/5(dout_4_1) -> Slice75/1
  [sync_out] -> vacc7/1(sync)
  [acc_len] -> vacc7/2(acc_len)
  [xxout4] -> reinterp_vacc24/1
  [yyout4] -> reinterp_vacc25/1
  [xyoutre4] -> reinterp_vacc26/1
  [xyoutim4] -> reinterp_vacc27/1
  [xxout5] -> reinterp_vacc28/1
  [yyout5] -> reinterp_vacc29/1
  [xyoutre5] -> reinterp_vacc30/1
  [xyoutim5] -> reinterp_vacc31/1
  vacc6/1(valid) -> Terminator19/1
  Slice68/1 -> [xx_vacc4]
  Slice69/1 -> [yy_vacc4]
  Slice70/1 -> [xy_vaccre4]
  Slice71/1 -> [xy_vaccim4]
  Slice72/1 -> [xx_vacc5]
  Slice73/1 -> [yy_vacc5]
  Slice74/1 -> [xy_vaccre5]
  Slice75/1 -> [xy_vaccim5]
  reinterp_vacc24/1 -> vacc6/3(din_1_1)
  reinterp_vacc25/1 -> vacc6/4(din_2_1)
  reinterp_vacc26/1 -> vacc6/5(din_3_1)
  reinterp_vacc27/1 -> vacc6/6(din_4_1)
  reinterp_vacc28/1 -> vacc7/3(din_1_1)
  reinterp_vacc29/1 -> vacc7/4(din_2_1)
  reinterp_vacc30/1 -> vacc7/5(din_3_1)
  reinterp_vacc31/1 -> vacc7/6(din_4_1)
  [xy_vaccre7] -> cram4/1(In1)
  [xy_vaccim7] -> cram4/2(In2)
  [xx_vacc7] -> cram4/3(In3)
  [yy_vacc7] -> cram4/4(In4)
  [xy_vaccre6] -> cram4/5(In5)
  [xy_vaccim6] -> cram4/6(In6)
  [xx_vacc6] -> cram4/7(In7)
  [yy_vacc6] -> cram4/8(In8)
  [xy_vaccre5] -> cram4/9(In9)
  [xy_vaccim5] -> cram4/10(In10)
  [xx_vacc5] -> cram4/11(In11)
  [yy_vacc5] -> cram4/12(In12)
  [xy_vaccre4] -> cram4/13(In13)
  [xy_vaccim4] -> cram4/14(In14)
  [xx_vacc4] -> cram4/15(In15)
  [yy_vacc4] -> cram4/16(In16)
  [xy_vaccre3] -> cram4/17(In17)
  [xy_vaccim3] -> cram4/18(In18)
  [xx_vacc3] -> cram4/19(In19)
  [yy_vacc3] -> cram4/20(In20)
  [xy_vaccre2] -> cram4/21(In21)
  [xy_vaccim2] -> cram4/22(In22)
  [xx_vacc2] -> cram4/23(In23)
  [yy_vacc2] -> cram4/24(In24)
  [xy_vaccre1] -> cram4/25(In25)
  [xy_vaccim1] -> cram4/26(In26)
  [xx_vacc1] -> cram4/27(In27)
  [yy_vacc1] -> cram4/28(In28)
  [xy_vaccre0] -> cram4/29(In29)
  [xy_vaccim0] -> cram4/30(In30)
  [xx_vacc0] -> cram4/31(In31)
  [yy_vacc0] -> cram4/32(In32)
  bus_expand/1(msb_out16) -> pfb0/2
  bus_expand/2(out15) -> pfb0/3
  bus_expand/3(out14) -> pfb0/4
  bus_expand/4(out13) -> pfb0/5
  bus_expand/5(out12) -> pfb0/6
  bus_expand/6(out11) -> pfb0/7
  bus_expand/7(out10) -> pfb0/8
  bus_expand/8(out9) -> pfb0/9
  bus_expand/9(out8) -> pfb0/10
  bus_expand/10(out7) -> pfb0/11
  bus_expand/11(out6) -> pfb0/12
  bus_expand/12(out5) -> pfb0/13
  bus_expand/13(out4) -> pfb0/14
  bus_expand/14(out3) -> pfb0/15
  bus_expand/15(out2) -> pfb0/16
  bus_expand/16(lsb_out1) -> pfb0/17
  bus_expand1/1(msb_out16) -> pfb1/2
  bus_expand1/2(out15) -> pfb1/3
  bus_expand1/3(out14) -> pfb1/4
  bus_expand1/4(out13) -> pfb1/5
  bus_expand1/5(out12) -> pfb1/6
  bus_expand1/6(out11) -> pfb1/7
  bus_expand1/7(out10) -> pfb1/8
  bus_expand1/8(out9) -> pfb1/9
  bus_expand1/9(out8) -> pfb1/10
  bus_expand1/10(out7) -> pfb1/11
  bus_expand1/11(out6) -> pfb1/12
  bus_expand1/12(out5) -> pfb1/13
  bus_expand1/13(out4) -> pfb1/14
  bus_expand1/14(out3) -> pfb1/15
  bus_expand1/15(out2) -> pfb1/16
  bus_expand1/16(lsb_out1) -> pfb1/17
  raw_adc_trig/1 -> Slice/1
  Slice/1 -> adcsnap0/3(trig), adcsnap1/3(trig)
  [adc_b] -> adcsnap1/1(din)
  [adc_a] -> adcsnap0/1(din)
  Constant17/1 -> adcsnap1/2(we)
  Constant13/1 -> adcsnap0/2(we)
  Slice40/1 -> Delay38/1
  Delay38/1 -> nspec/1
  [spec_cntr] -> Slice40/1
  bus_create/1(bus_out) -> Delay39/1, Delay40/1
  Delay39/1 -> status/1
  Delay40/1 -> debug/1(din)
  Delay41/1 -> debug/2(we)
  [data_valid] -> Delay41/1
  [xxout0] -> stokesout/1(din)
  [sync_out] -> stokesout/2(we), stokesout/3(trig)
  [xx_vacc0] -> Delay58/1
  [valid] -> vaccout/3(trig), Delay62/1
  Slice41/1 -> Delay63/1
  [master_rst1] -> Register/1
  Register/1 -> ssg/1(reset)
  [spec_tick] -> ssg/2(spec_tick)
  fft1/10 -> Terminator30/1
  fft1/9 -> Register8/1
  fft1/8 -> Register7/1
  fft1/7 -> Register15/1
  fft1/6 -> Register14/1
  fft1/5 -> Register13/1
  [master_rst1] -> pkt_cntr1/1
  [eof] -> pkt_cntr1/2
  pkt_cntr1/1 -> Delay59/1
  Delay59/1 -> npkt/1
  edge_detect5/1 -> [adc_sync]
  edge_detect4/1(out) -> Delay61/1
  Delay60/1 -> Logical2/2
  [time_cntr] -> timesnap/1(din)
  edge_detect/1 -> timesnap/2(we)
  [master_rst1] -> timesnap/3(trig)
  Mux/1 -> edge_detect/1
  [sync_out] -> Mux/2
  [valid] -> Mux/3
  [data_valid_spead] -> Mux/5
  [data_valid] -> Mux/4
  Convert1/1 -> Mux/1, Register17/1
  Delay58/1 -> vaccout/1(din)
  Delay62/1 -> vaccout/2(we)
  Delay63/1 -> rshpout/1(din)
  Delay64/1 -> rshpout/2(we)
  Constant1/1 -> timesel/1
  timesel/1 -> Convert1/1
  fftshift/1 -> [fftshift]
  Constant2/1 -> fftshift/1
  pfb1/1 -> fft1/1
  pfb1/2 -> fft1/3
  pfb1/3 -> fft1/4
  pfb1/4 -> fft1/5
  pfb1/5 -> fft1/6
  pfb1/6 -> fft1/7
  pfb1/7 -> fft1/8
  pfb1/8 -> fft1/9
  pfb1/9 -> fft1/10
  pfb1/10 -> fft1/11
  pfb1/11 -> fft1/12
  pfb1/12 -> fft1/13
  pfb1/13 -> fft1/14
  pfb1/14 -> fft1/15
  pfb1/15 -> fft1/16
  pfb1/16 -> fft1/17
  pfb1/17 -> fft1/18
  pfb0/1 -> fft0/1
  pfb0/2 -> fft0/3
  pfb0/3 -> fft0/4
  pfb0/4 -> fft0/5
  pfb0/5 -> fft0/6
  pfb0/6 -> fft0/7
  pfb0/7 -> fft0/8
  pfb0/8 -> fft0/9
  pfb0/9 -> fft0/10
  pfb0/10 -> fft0/11
  pfb0/11 -> fft0/12
  pfb0/12 -> fft0/13
  pfb0/13 -> fft0/14
  pfb0/14 -> fft0/15
  pfb0/15 -> fft0/16
  pfb0/16 -> fft0/17
  pfb0/17 -> fft0/18
  [adc_a] -> bus_expand/1(bus_in)
  [adc_b] -> bus_expand1/1(bus_in)
  Delay2/1 -> [sync_out]
  Logical1/1 -> reshaper/1(rst), [rshp_rst]
  [data_enable] -> Logical1/1
  Delay61/1 -> Logical1/2
  sync_delay/1 -> Register19/3
  Mux1/1 -> edge_detect6/1
  edge_detect6/1 -> debug/3(trig)
  [rshp_rst] -> bus_create/1(in1)
  [data_valid] -> bus_create/2(in2)
  [eoh] -> bus_create/3(in3)
  [eof] -> bus_create/4(in4)
  [spead_st] -> bus_create/5(in5)
  [data_valid_spead] -> bus_create/6(in6)
  [reshape_state] -> bus_create/7(in7)
  [data_enable] -> bus_create/8(in8)
  [spead_rst] -> bus_create/9(in9)
  [master_rst1] -> bus_create/10(in10)
  [tge_rst] -> bus_create/11(in11)
  [valid] -> bus_create/12(in12)
  [txovf] -> bus_create/13(in13)
  [data_enable] -> Mux1/2
  [spead_rst] -> Mux1/3
  [ext_pps_en] -> Mux1/5
  [master_rst1] -> Mux1/4
  [trigsel] -> Mux1/1
  sg/3(ext_pps_en) -> [ext_pps_en]
  Register17/1 -> [trigsel]
  [data_enable] -> Logical4/1
  [data_valid] -> speader1/2(valid_in)
  [data_valid_spead] -> Logical4/2
  Logical4/1 -> Delay25/1
